simulink model "TosAeb"  (R2021a)
settings: Solver=FixedStepDiscrete  StartTime=0.0  StopTime=inf  FixedStep=0.01  RelTol=1e-3
undefined symbols (not defined in the file or in its folder): ACCModSts, AccSts1, AEB_ObjectMovingOrientation, AEB_ObjectMovingState, CN_BTCActive, CN_CurrentGearR, CN_CurrentGearR_DHT, CN_PTCActive, CN_VDCActive, CN_VSC_EspOff, CN_VSC_FCW_AEB_Error, CN_VSC_FCW_AEB_NoDispay, CN_VSC_FCW_AEB_PerfmDgdtn, CN_VSC_FCW_AEB_TempUnAvlbl, CN_VSC_VehStandstill, CNaccelAvoidMax, CNaccelAvoidMin, CNbrakePedalPress, CNcomfortStrLeftAccelMax, CNcomfortStrLeftAccelMin, CNcomfortStrRightAccelMax, CNcomfortStrRightAccelMin, CNDrvDoorOpen, CNDrvModeEco, CNDrvModeNorm, CNDrvModeOther, CNDrvModeSport, CNDrvSBRreminder, CNNull, CNobjInvalidID, CNPassDoorOpen, CNPassSBRreminder, CNPEBSAccelNegInf, CNPEBSAccelNull, CNPEBSTimeInf, CNPEBSTimeNull, CNSTOPMAX, CNSTOPNULL, CNsubjWidth, CNSwtOFF, CNSwtON, CNTTCMAX, CNTTCNULL, CNVehConfigAEBCycNone, CNVehConfigAEBNone, CNVehConfigAEBPedNone, CNVehConfigFCWNone, CNVehConfigJANone, CollisionSeverity, FALSE, FCWSnvty, JACycObjCalcInfoDefaultParams, JAPedObjCalcInfoDefaultParams, JAVehObjCalcInfoDefaultParams, latCycObjCalcInfoDefaultParams, latPedObjCalcInfoDefaultParams, longObjCalcInfoDefaultParams, ModSts, movingStatus, ObjectType ... (+1112)
libraries: simulink x3031, AEBSFunctionLib x50, Ta_Library x33, AEBSStateMachineLib x23, Mylibrary x12, simulink_extras x7, ObjSelect_lib x3, dspstat3 x2
(part 17 of 102)

== system TosAeb/FcwAebJa_Step_sys/ALG_AEBSystem/ALG_AEBController/AEB_StateMachineCalcApp/JaFctStsArb/BrkArb ==
  JA_vehAEBModSts : Inport
  JA_pedAEBModSts : Inport  Port=2
  JA_cycAEBModSts : Inport  Port=3
  JA_PTW_AEBModSts : Inport  Port=4
  BrkAborArb : Subsystem
  Bus Creator : BusCreator  Inputs=8
  Bus Creator1 : BusCreator  Inputs=4
  Bus Selector : BusSelector  OutputSignals=JA_vehAEBModSts,JA_pedAEBModSts,JA_cycAEBModSts,JA_PTW_AEBModSts
  Bus Selector1 : BusSelector  OutputSignals=JA_vehAEBModSts,JA_pedAEBModSts,JA_cycAEBModSts,JA_PTW_AEBModSts
  Bus Selector2 : BusSelector  OutputSignals=JA_vehAEBModSts,JA_PTW_AEBModSts,JA_cycAEBModSts,JA_pedAEBModSts
  JA_BrkActvArb : Subsystem
  JA_BrkModStsArbtrtn : Subsystem
  JA_BrkSuppArb : Subsystem
  JA_vehAEBModStsArb : Outport
  JA_PTW_AEBModStsArb : Outport  Port=2
  JA_cycAEBModStsArb : Outport  Port=3
  JA_pedAEBModStsArb : Outport  Port=4
  brkSuppAborCondArb : Outport  Port=5
  brkActvCond : Outport  Port=6
  wires:
  JA_BrkModStsArbtrtn/3(JA_cycAEBModStsArb) -> [JA_cycAEBModStsArb], JA_BrkActvArb/3(JA_cycAEBModStsArb)
  JA_BrkActvArb/1(JA_BrkActvCond) -> brkActvCond/1
  Bus Creator/1 -> brkSuppAborCondArb/1
  JA_BrkModStsArbtrtn/1(JA_vehAEBModStsArb) -> [JA_vehAEBModStsArb], JA_BrkActvArb/1(JA_vehAEBModStsArb)
  JA_BrkModStsArbtrtn/2(JA_PTW_AEBModStsArb) -> [JA_PTW_AEBModStsArb], JA_BrkActvArb/2(JA_PTW_AEBModStsArb)
  Bus Selector/1 -> JA_BrkSuppArb/1(JA_vehAEBModSts)  "<JA_vehAEBModSts>"
  Bus Selector/2 -> JA_BrkSuppArb/2(JA_pedAEBModSts)  "<JA_pedAEBModSts>"
  Bus Selector/3 -> JA_BrkSuppArb/3(JA_cycAEBModSts)  "<JA_cycAEBModSts>"
  Bus Selector/4 -> JA_BrkSuppArb/4(JA_PTW_AEBModSts)  "<JA_PTW_AEBModSts>"
  JA_PTW_AEBModSts/1 -> Bus Creator1/4  "JA_PTW_AEBModSts"
  JA_cycAEBModSts/1 -> Bus Creator1/3  "JA_cycAEBModSts"
  JA_pedAEBModSts/1 -> Bus Creator1/2  "JA_pedAEBModSts"
  JA_vehAEBModSts/1 -> Bus Creator1/1  "JA_vehAEBModSts"
  BrkAborArb/1(JA_vehAEBAborArb) -> Bus Creator/5, [JA_vehAEBAborArb]  "JA_vehAEBAborArb"
  BrkAborArb/2(JA_PTW_AEBAborArb) -> Bus Creator/6, [JA_PTW_AEBAborArb]  "JA_PTW_AEBAborArb"
  BrkAborArb/3(JA_cycAEBAborArb) -> Bus Creator/7, [JA_cycAEBAborArb]  "JA_cycAEBAborArb"
  BrkAborArb/4(JA_pedAEBAborArb) -> [JA_pedAEBAborArb], Bus Creator/8  "JA_pedAEBAborArb"
  Bus Selector1/1 -> BrkAborArb/1(JA_vehAEBModSts)  "<JA_vehAEBModSts>"
  Bus Selector1/2 -> BrkAborArb/2(JA_pedAEBModSts)  "<JA_pedAEBModSts>"
  Bus Selector1/3 -> BrkAborArb/3(JA_cycAEBModSts)  "<JA_cycAEBModSts>"
  Bus Selector1/4 -> BrkAborArb/4(JA_PTW_AEBModSts)  "<JA_PTW_AEBModSts>"
  JA_BrkSuppArb/1(JA_vehAEBSuppArb) -> Bus Creator/1  "JA_vehAEBSuppArb"
  JA_BrkSuppArb/2(JA_PTW_AEBSuppArb) -> Bus Creator/2  "JA_PTW_AEBSuppArb"
  JA_BrkSuppArb/3(JA_cycAEBSuppArb) -> Bus Creator/3  "JA_cycAEBSuppArb"
  JA_BrkSuppArb/4(JA_pedAEBSuppArb) -> Bus Creator/4  "JA_pedAEBSuppArb"
  [JA_vehAEBAborArb] -> JA_BrkModStsArbtrtn/5(JA_vehAEBAborArb)
  [JA_PTW_AEBAborArb] -> JA_BrkModStsArbtrtn/6(JA_PTW_AEBAborArb)
  [JA_cycAEBAborArb] -> JA_BrkModStsArbtrtn/7(JA_cycAEBAborArb)
  [JA_pedAEBAborArb] -> JA_BrkModStsArbtrtn/8(JA_pedAEBAborArb)
  Bus Creator1/1 -> Bus Selector2/1, Bus Selector1/1, Bus Selector/1
  JA_BrkModStsArbtrtn/4(JA_pedAEBModStsArb) -> JA_BrkActvArb/4(JA_pedAEBModStsArb), [JA_pedAEBModStsArb]
  [JA_vehAEBModStsArb] -> JA_vehAEBModStsArb/1
  [JA_PTW_AEBModStsArb] -> JA_PTW_AEBModStsArb/1
  [JA_pedAEBModStsArb] -> JA_pedAEBModStsArb/1
  [JA_cycAEBModStsArb] -> JA_cycAEBModStsArb/1
  Bus Selector2/1 -> JA_BrkModStsArbtrtn/1(JA_vehAEBModSts)  "<JA_vehAEBModSts>"
  Bus Selector2/2 -> JA_BrkModStsArbtrtn/2(JA_PTW_AEBModSts)  "<JA_PTW_AEBModSts>"
  Bus Selector2/3 -> JA_BrkModStsArbtrtn/3(JA_cycAEBModSts)  "<JA_cycAEBModSts>"
  Bus Selector2/4 -> JA_BrkModStsArbtrtn/4(JA_pedAEBModSts)  "<JA_pedAEBModSts>"

== system TosAeb/FcwAebJa_Step_sys/ALG_AEBSystem/ALG_AEBController/AEB_StateMachineCalcApp/JaFctStsArb/BrkReadyArb ==
  brkActvCond : Inport
  JA_vehPrefillModSts : Inport  Port=2
  JA_pedPrefillModSts : Inport  Port=3
  JA_cycPrefillModSts : Inport  Port=4
  JA_PTW_PrefillModSts : Inport  Port=5
  BrkAborArb : Subsystem
  BrkReadyActvArb : Subsystem
  BrkReadyModStsArbtrtn : Subsystem
  BrkReadySuppArb : Subsystem
  Bus Creator : BusCreator  Inputs=8
  Bus Creator1 : BusCreator  Inputs=5
  Bus Selector : BusSelector  OutputSignals=JA_vehPrefillModSts,JA_pedPrefillModSts,JA_cycPrefillModSts,JA_PTW_PrefillModSts,brkActvCond
  Bus Selector1 : BusSelector  OutputSignals=JA_vehPrefillModSts,JA_pedPrefillModSts,JA_cycPrefillModSts,JA_PTW_PrefillModSts,brkActvCond
  Bus Selector2 : BusSelector  OutputSignals=JA_vehPrefillModSts,JA_PTW_PrefillModSts,JA_cycPrefillModSts,JA_pedPrefillModSts
  Terminator : Terminator
  brkReadySuppAborCondArb : Outport
  JA_vehPrefillModStsArb : Outport  Port=2
  JA_PTW_PrefillModStsArb : Outport  Port=3
  JA_cycPrefillModStsArb : Outport  Port=4
  JA_pedPrefillModStsArb : Outport  Port=5
  wires:
  [JA_cycPrefillModStsArb] -> JA_cycPrefillModStsArb/1
  [JA_pedPrefillModStsArb] -> JA_pedPrefillModStsArb/1
  [JA_PTW_PrefillModStsArb] -> JA_PTW_PrefillModStsArb/1
  [JA_vehPrefillModStsArb] -> JA_vehPrefillModStsArb/1
  BrkReadyModStsArbtrtn/4(JA_pedPrefillModStsArb) -> BrkReadyActvArb/4(JA_pedPrefillModStsArb), [JA_pedPrefillModStsArb]
  Bus Creator1/1 -> Bus Selector/1, Bus Selector1/1, Bus Selector2/1
  Bus Selector2/1 -> BrkReadyModStsArbtrtn/1(JA_vehPrefillModSts)  "<JA_vehPrefillModSts>"
  Bus Selector2/3 -> BrkReadyModStsArbtrtn/3(JA_cycPrefillModSts)  "<JA_cycPrefillModSts>"
  [JA_pedPrefillAborArb] -> BrkReadyModStsArbtrtn/8(JA_pedPrefillAborArb)
  [JA_cycPrefillAborArb] -> BrkReadyModStsArbtrtn/7(JA_cycPrefillAborArb)
  [JA_PTW_PrefillAborArb] -> BrkReadyModStsArbtrtn/6(JA_PTW_PrefillAborArb)
  [JA_vehPrefillAborArb] -> BrkReadyModStsArbtrtn/5(JA_vehPrefillAborArb)
  BrkReadySuppArb/4(JA_pedPrefillSuppArb) -> Bus Creator/4  "JA_pedPrefillSuppArb"
  BrkReadySuppArb/3(JA_cycPrefillSuppArb) -> Bus Creator/3  "JA_cycPrefillSuppArb"
  BrkReadySuppArb/2(JA_PTW_PrefillSuppArb) -> Bus Creator/2  "JA_PTW_PrefillSuppArb"
  BrkReadySuppArb/1(JA_vehPrefillSuppArb) -> Bus Creator/1  "JA_vehPrefillSuppArb"
  Bus Selector1/4 -> BrkAborArb/4(JA_PTW_PrefillModSts)  "<JA_PTW_PrefillModSts>"
  Bus Selector1/3 -> BrkAborArb/3(JA_cycPrefillModSts)  "<JA_cycPrefillModSts>"
  Bus Selector1/2 -> BrkAborArb/2(JA_pedPrefillModSts)  "<JA_pedPrefillModSts>"
  Bus Selector1/1 -> BrkAborArb/1(JA_vehPrefillModSts)  "<JA_vehPrefillModSts>"
  BrkAborArb/4(JA_pedPrefillAborArb) -> Bus Creator/8, [JA_pedPrefillAborArb]  "JA_pedPrefillAborArb"
  BrkAborArb/3(JA_cycPrefillAborArb) -> [JA_cycPrefillAborArb], Bus Creator/7  "JA_cycPrefillAborArb"
  BrkAborArb/2(JA_PTW_PrefillAborArb) -> [JA_PTW_PrefillAborArb], Bus Creator/6  "JA_PTW_PrefillAborArb"
  BrkAborArb/1(JA_vehPrefillAborArb) -> [JA_vehPrefillAborArb], Bus Creator/5  "JA_vehPrefillAborArb"
  JA_vehPrefillModSts/1 -> Bus Creator1/1  "JA_vehPrefillModSts"
  JA_pedPrefillModSts/1 -> Bus Creator1/2  "JA_pedPrefillModSts"
  JA_cycPrefillModSts/1 -> Bus Creator1/3  "JA_cycPrefillModSts"
  JA_PTW_PrefillModSts/1 -> Bus Creator1/4  "JA_PTW_PrefillModSts"
  Bus Selector/5 -> BrkReadySuppArb/5(JA_BrkActvCond)  "<brkActvCond>"
  Bus Selector/4 -> BrkReadySuppArb/4(JA_PTW_PrefillModSts)  "<JA_PTW_PrefillModSts>"
  Bus Selector/3 -> BrkReadySuppArb/3(JA_cycPrefillModSts)  "<JA_cycPrefillModSts>"
  Bus Selector/2 -> BrkReadySuppArb/2(JA_pedPrefillModSts)  "<JA_pedPrefillModSts>"
  Bus Selector/1 -> BrkReadySuppArb/1(JA_vehPrefillModSts)  "<JA_vehPrefillModSts>"
  BrkReadyModStsArbtrtn/2(JA_PTW_PrefillModStsArb) -> BrkReadyActvArb/2(JA_PTW_PrefillModStsArb), [JA_PTW_PrefillModStsArb]
  BrkReadyModStsArbtrtn/1(JA_vehPrefillModStsArb) -> BrkReadyActvArb/1(JA_vehPrefillModStsArb), [JA_vehPrefillModStsArb]
  Bus Creator/1 -> brkReadySuppAborCondArb/1
  BrkReadyModStsArbtrtn/3(JA_cycPrefillModStsArb) -> BrkReadyActvArb/3(JA_cycPrefillModStsArb), [JA_cycPrefillModStsArb]
  brkActvCond/1 -> Bus Creator1/5  "brkActvCond"
  Bus Selector1/5 -> BrkAborArb/5(JA_BrkActvCond)  "<brkActvCond>"
  BrkReadyActvArb/1(JA_BrkActvCond) -> Terminator/1
  Bus Selector2/4 -> BrkReadyModStsArbtrtn/4(JA_pedPrefillModSts)  "<JA_pedPrefillModSts>"
  Bus Selector2/2 -> BrkReadyModStsArbtrtn/2(JA_PTW_PrefillModSts)  "<JA_PTW_PrefillModSts>"

== system TosAeb/FcwAebJa_Step_sys/ALG_AEBSystem/ALG_AEBController/AEB_StateMachineCalcApp/LatAEBStateMachine/cycAEBfullStateMachine ==
  inputSignal : Inport
  AEBFuncFaultMap : Subsystem
  AEBStateMachine : AEBSStateMachineLib/AEBStateMachine  SystemSampleTime=-1  VariantActivationTime=update diagram  Latency=0  AutoFrameSizeCalculation=off
  ActivationCondCalc : Subsystem
  ActiveStateAborCondCalc : Subsystem
  Bus Selector : BusSelector  OutputSignals=AVHStsActv,vehStateCalcInfo.VSC_FCW_AEB_FctSts.FCW_AEB_PedFctSts,vehStateCalcInfo.VSC_AEBFuncSwtSts.AEB_PedResp,vehStateCalcInfo.VSCVehStandStill
  Bus Selector1 : BusSelector  OutputSignals=CycFuncSuppAborCond.cycAEBfullSuppCond,latFctStsArbSuppAborCond.brkSuppAborCondArb.cycAEBfullSuppArb,FuncImpSuppAborCond.brkFuncImpSuppAborCond.brkFuncImpSuppCond.cycAEBfullFuncImpSuppCond
  Bus Selector2 : BusSelector  OutputSignals=CycFuncSuppAborCond.cycAEBfullAborCondNoStandstill,latFctStsArbSuppAborCond.brkSuppAborCondArb.cycAEBfullAborArb,FuncImpSuppAborCond.brkFuncImpSuppAborCond.brkFuncImpAborCond.cycAEBfullFuncImpAborCond,FuncManeuAborCond.latBrkManeuAborCond
  Bus Selector3 : BusSelector  OutputSignals=CycFuncSuppAborCond.cycAEBfullAborCondStandstill,latFctStsArbSuppAborCond.brkSuppAborCondArb.cycAEBfullAborArb,FuncImpSuppAborCond.brkFuncImpSuppAborCond.brkFuncImpAborCond.cycAEBfullFuncImpAborCond,FuncManeuAborCond.latBrkManeuAborCond
  Constant : Constant  Value=5
  Constant1 : Constant  Value=P_AEB_FuncStatus_CycAEBfullStandstillWorkTime
  Constant2 : Constant  Value=Ts
  StandstillAborCondCalc : Subsystem
  cycAEBfullRawModSts : Outport
  cycAEBfullModSts : Outport  Port=2
  cycAEBfullFuncActiveTime : Outport  Port=3
  wires:
  inputSignal/1 -> Bus Selector3/1, Bus Selector2/1, Bus Selector1/1, Bus Selector/1
  Bus Selector2/2 -> ActiveStateAborCondCalc/2(cycAEBfullAborArb)  "<cycAEBfullAborArb>"
  Bus Selector2/3 -> ActiveStateAborCondCalc/3(cycAEBfullFuncImpAborCond)  "<cycAEBfullFuncImpAborCond>"
  Bus Selector1/2 -> ActivationCondCalc/2(cycAEBfullSuppArb)  "<cycAEBfullSuppArb>"
  Bus Selector1/3 -> ActivationCondCalc/3(cycAEBfullFuncImpSuppCond)  "<cycAEBfullFuncImpSuppCond>"
  Bus Selector2/1 -> ActiveStateAborCondCalc/1(cycAEBfullAborCondNoStandstill)  "<cycAEBfullAborCondNoStandstill>"
  Bus Selector1/1 -> ActivationCondCalc/1(cycAEBfullSuppCond)  "<cycAEBfullSuppCond>"
  Bus Selector2/4 -> ActiveStateAborCondCalc/4(latBrkManeuAborCond)  "<latBrkManeuAborCond>"
  Bus Selector3/1 -> StandstillAborCondCalc/1(cycAEBfullAborCondStandstill)  "<cycAEBfullAborCondStandstill>"
  Bus Selector3/2 -> StandstillAborCondCalc/2(cycAEBfullAborArb)  "<cycAEBfullAborArb>"
  Bus Selector3/3 -> StandstillAborCondCalc/3(cycAEBfullFuncImpAborCond)  "<cycAEBfullFuncImpAborCond>"
  Bus Selector3/4 -> StandstillAborCondCalc/4(latBrkManeuAborCond)  "<latBrkManeuAborCond>"
  AEBStateMachine/3 -> cycAEBfullFuncActiveTime/1  "cycAEBfullFuncActiveTime"
  AEBStateMachine/2 -> cycAEBfullModSts/1  "cycAEBfullModSts"
  AEBStateMachine/1 -> cycAEBfullRawModSts/1
  Constant2/1 -> AEBStateMachine/8
  Constant/1 -> AEBStateMachine/9
  Constant1/1 -> AEBStateMachine/10
  Bus Selector/2 -> AEBFuncFaultMap/1(FCW_AEB_VehFctSts)  "<FCW_AEB_PedFctSts>"
  Bus Selector/3 -> AEBStateMachine/3  "<AEB_PedResp>"
  Bus Selector/1 -> AEBStateMachine/1  "<AVHStsActv>"
  Bus Selector/4 -> AEBStateMachine/4  "<VSCVehStandStill>"
  ActivationCondCalc/1(ActivationCondFulfilled) -> AEBStateMachine/5
  ActiveStateAborCondCalc/1(ActiveStateAborCondFulfilled) -> AEBStateMachine/6
  StandstillAborCondCalc/1(ActiveStateAborCondFulfilled) -> AEBStateMachine/7
  AEBFuncFaultMap/1(Fault) -> AEBStateMachine/2

== system TosAeb/FcwAebJa_Step_sys/ALG_AEBSystem/ALG_AEBController/AEB_StateMachineCalcApp/LatAEBStateMachine/cycAEBpartStateMachine ==
  inputSignal : Inport
  AEBFuncFaultMap : Subsystem
  AEBStateMachine : AEBSStateMachineLib/AEBStateMachine  SystemSampleTime=-1  VariantActivationTime=update diagram  Latency=0  AutoFrameSizeCalculation=off
  ActivationCondCalc : Subsystem
  ActiveStateAborCondCalc : Subsystem
  Bus Selector : BusSelector  OutputSignals=AVHStsActv,vehStateCalcInfo.VSC_FCW_AEB_FctSts.FCW_AEB_PedFctSts,vehStateCalcInfo.VSC_AEBFuncSwtSts.AEB_PedResp,vehStateCalcInfo.VSCVehStandStill
  Bus Selector1 : BusSelector  OutputSignals=CycFuncSuppAborCond.cycAEBpartSuppCond,latFctStsArbSuppAborCond.brkSuppAborCondArb.cycAEBpartSuppArb,FuncImpSuppAborCond.brkFuncImpSuppAborCond.brkFuncImpSuppCond.cycAEBpartFuncImpSuppCond
  Bus Selector2 : BusSelector  OutputSignals=CycFuncSuppAborCond.cycAEBpartAborCondNoStandstill,latFctStsArbSuppAborCond.brkSuppAborCondArb.cycAEBpartAborArb,FuncImpSuppAborCond.brkFuncImpSuppAborCond.brkFuncImpAborCond.cycAEBpartFuncImpAborCond,FuncManeuAborCond.latBrkManeuAborCond
  Bus Selector3 : BusSelector  OutputSignals=CycFuncSuppAborCond.cycAEBpartAborCondStandstill,latFctStsArbSuppAborCond.brkSuppAborCondArb.cycAEBpartAborArb,FuncImpSuppAborCond.brkFuncImpSuppAborCond.brkFuncImpAborCond.cycAEBpartFuncImpAborCond,FuncManeuAborCond.latBrkManeuAborCond
  Constant : Constant  Value=5
  Constant1 : Constant  Value=P_AEB_FuncStatus_CycAEBpartStandstillWorkTime
  Constant2 : Constant  Value=Ts
  StandstillAborCondCalc : Subsystem
  cycAEBpartRawModSts : Outport
  cycAEBpartModSts : Outport  Port=2
  cycAEBpartFuncActiveTime : Outport  Port=3
  wires:
  inputSignal/1 -> Bus Selector3/1, Bus Selector2/1, Bus Selector1/1, Bus Selector/1
  Bus Selector2/2 -> ActiveStateAborCondCalc/2(cycAEBpartAborArb)  "<cycAEBpartAborArb>"
  Bus Selector2/3 -> ActiveStateAborCondCalc/3(cycAEBpartFuncImpAborCond)  "<cycAEBpartFuncImpAborCond>"
  Bus Selector1/2 -> ActivationCondCalc/2(cycAEBpartSuppArb)  "<cycAEBpartSuppArb>"
  Bus Selector1/3 -> ActivationCondCalc/3(cycAEBpartFuncImpSuppCond)  "<cycAEBpartFuncImpSuppCond>"
  Bus Selector2/1 -> ActiveStateAborCondCalc/1(cycAEBpartAborCondNoStandstill)  "<cycAEBpartAborCondNoStandstill>"
  Bus Selector1/1 -> ActivationCondCalc/1(cycAEBpartSuppCond)  "<cycAEBpartSuppCond>"
  Bus Selector2/4 -> ActiveStateAborCondCalc/4(latBrkManeuAborCond)  "<latBrkManeuAborCond>"
  Bus Selector3/1 -> StandstillAborCondCalc/1(cycAEBpartAborCondStandstill)  "<cycAEBpartAborCondStandstill>"
  Bus Selector3/2 -> StandstillAborCondCalc/2(cycAEBpartAborArb)  "<cycAEBpartAborArb>"
  Bus Selector3/3 -> StandstillAborCondCalc/3(cycAEBpartFuncImpAborCond)  "<cycAEBpartFuncImpAborCond>"
  Bus Selector3/4 -> StandstillAborCondCalc/4(latBrkManeuAborCond)  "<latBrkManeuAborCond>"
  AEBStateMachine/3 -> cycAEBpartFuncActiveTime/1  "cycAEBpartFuncActiveTime"
  AEBStateMachine/2 -> cycAEBpartModSts/1  "cycAEBpartModSts"
  AEBStateMachine/1 -> cycAEBpartRawModSts/1
  Bus Selector/2 -> AEBFuncFaultMap/1(FCW_AEB_VehFctSts)  "<FCW_AEB_PedFctSts>"
  Bus Selector/3 -> AEBStateMachine/3  "<AEB_PedResp>"
  Bus Selector/1 -> AEBStateMachine/1  "<AVHStsActv>"
  Bus Selector/4 -> AEBStateMachine/4  "<VSCVehStandStill>"
  Constant2/1 -> AEBStateMachine/8
  Constant/1 -> AEBStateMachine/9
  Constant1/1 -> AEBStateMachine/10
  ActivationCondCalc/1(ActivationCondFulfilled) -> AEBStateMachine/5
  ActiveStateAborCondCalc/1(ActiveStateAborCondFulfilled) -> AEBStateMachine/6
  StandstillAborCondCalc/1(ActiveStateAborCondFulfilled) -> AEBStateMachine/7
  AEBFuncFaultMap/1(Fault) -> AEBStateMachine/2

== system TosAeb/FcwAebJa_Step_sys/ALG_AEBSystem/ALG_AEBController/AEB_StateMachineCalcApp/LatAEBStateMachine/cycPrefillStateMachine ==
  inputSignal : Inport
  AEBFuncFaultMap : Subsystem
  AEBStateMachine : AEBSStateMachineLib/AEBStateMachine  SystemSampleTime=-1  VariantActivationTime=update diagram  Latency=0  AutoFrameSizeCalculation=off
  ActivationCondCalc : Subsystem
  ActiveStateAborCondCalc : Subsystem
  Bus Selector : BusSelector  OutputSignals=vehStateCalcInfo.VSC_FCW_AEB_FctSts.FCW_AEB_PedFctSts,vehStateCalcInfo.VSC_AEBFuncSwtSts.AEB_PedResp
  Bus Selector1 : BusSelector  OutputSignals=CycFuncSuppAborCond.cycPrefillSuppCond,latFctStsArbSuppAborCond.brkReadySuppAborCond.cycPrefillSuppArb,FuncImpSuppAborCond.brkReadyFuncImpSuppAborCond.brkReadyFuncImpSuppCond.cycPrefillFuncImpSuppCond
  Bus Selector2 : BusSelector  OutputSignals=CycFuncSuppAborCond.cycPrefillAborCond,latFctStsArbSuppAborCond.brkReadySuppAborCond.cycPrefillAborArb,FuncImpSuppAborCond.brkReadyFuncImpSuppAborCond.brkReadyFuncImpAborCond.cycPrefillFuncImpAborCond,FuncManeuAborCond.ABPFuncManeuAborCond
  Constant : Constant  Value=5
  Constant1 : Constant  Value=P_AEB_FuncStatus_CycPrefillStandstillWorkTime
  Constant2 : Constant  Value=Ts
  Constant3 : Constant  Value=FALSE
  Constant4 : Constant  Value=FALSE
  Constant5 : Constant  Value=FALSE
  cycPrefillRawModSts : Outport
  cycPrefillModSts : Outport  Port=2
  cycPrefillFuncActiveTime : Outport  Port=3
  wires:
  Bus Selector1/2 -> ActivationCondCalc/2(cycPrefillSuppArb)  "<cycPrefillSuppArb>"
  Bus Selector1/1 -> ActivationCondCalc/1(cycPrefillSuppCond)  "<cycPrefillSuppCond>"
  Bus Selector2/1 -> ActiveStateAborCondCalc/1(cycPrefillAborCond)  "<cycPrefillAborCond>"
  Bus Selector1/3 -> ActivationCondCalc/3(cycPrefillFuncImpSuppCond)  "<cycPrefillFuncImpSuppCond>"
  Bus Selector2/3 -> ActiveStateAborCondCalc/3(cycPrefillFuncImpAborCond)  "<cycPrefillFuncImpAborCond>"
  Bus Selector2/4 -> ActiveStateAborCondCalc/4(ABPFuncManeuAborCond)  "<ABPFuncManeuAborCond>"
  Bus Selector2/2 -> ActiveStateAborCondCalc/2(cycPrefillAborArb)  "<cycPrefillAborArb>"
  inputSignal/1 -> Bus Selector2/1, Bus Selector1/1, Bus Selector/1
  AEBStateMachine/3 -> cycPrefillFuncActiveTime/1  "cycPrefillFuncActiveTime"
  AEBStateMachine/2 -> cycPrefillModSts/1  "cycPrefillModSts"
  AEBStateMachine/1 -> cycPrefillRawModSts/1
  Constant5/1 -> AEBStateMachine/1
  Constant4/1 -> AEBStateMachine/4
  Bus Selector/1 -> AEBFuncFaultMap/1(FCW_AEB_VehFctSts)  "<FCW_AEB_PedFctSts>"
  Bus Selector/2 -> AEBStateMachine/3  "<AEB_PedResp>"
  Constant3/1 -> AEBStateMachine/7
  Constant2/1 -> AEBStateMachine/8
  Constant/1 -> AEBStateMachine/9
  Constant1/1 -> AEBStateMachine/10
  ActivationCondCalc/1(ActivationCondFulfilled) -> AEBStateMachine/5
  ActiveStateAborCondCalc/1(ActiveStateAborCondFulfilled) -> AEBStateMachine/6
  AEBFuncFaultMap/1(Fault) -> AEBStateMachine/2

== system TosAeb/FcwAebJa_Step_sys/ALG_AEBSystem/ALG_AEBController/AEB_StateMachineCalcApp/LatAEBStateMachine/pedABAStateMachine ==
  inputSignal : Inport
  AEBFuncFaultMap : Subsystem
  AEBStateMachine : AEBSStateMachineLib/AEBStateMachine  SystemSampleTime=-1  VariantActivationTime=update diagram  Latency=0  AutoFrameSizeCalculation=off
  ActivationCondCalc : Subsystem
  ActiveStateAborCondCalc : Subsystem
  Bus Selector : BusSelector  OutputSignals=vehStateCalcInfo.VSC_FCW_AEB_FctSts.FCW_AEB_PedFctSts,vehStateCalcInfo.VSC_AEBFuncSwtSts.AEB_PedResp
  Bus Selector1 : BusSelector  OutputSignals=PedFuncSuppAborCond.pedABASuppCond,latFctStsArbSuppAborCond.brkReadySuppAborCond.pedABASuppArb,FuncImpSuppAborCond.brkReadyFuncImpSuppAborCond.brkReadyFuncImpSuppCond.pedABAFuncImpSuppCond
  Bus Selector2 : BusSelector  OutputSignals=PedFuncSuppAborCond.pedABAAborCond,latFctStsArbSuppAborCond.brkReadySuppAborCond.pedABAAborArb,FuncImpSuppAborCond.brkReadyFuncImpSuppAborCond.brkReadyFuncImpAborCond.pedABAFuncImpAborCond,FuncManeuAborCond.ABAFuncManeuAborCond
  Constant : Constant  Value=256
  Constant1 : Constant  Value=P_AEB_FuncStatus_PedABAStandstillWorkTime
  Constant2 : Constant  Value=Ts
  Constant3 : Constant  Value=FALSE
  Constant4 : Constant  Value=FALSE
  Constant5 : Constant  Value=FALSE
  pedABARawModSts : Outport
  pedABAModSts : Outport  Port=2
  pedABAFuncActiveTime : Outport  Port=3
  wires:
  Bus Selector1/2 -> ActivationCondCalc/2(pedABASuppArb)  "<pedABASuppArb>"
  Bus Selector1/1 -> ActivationCondCalc/1(pedABASuppCond)  "<pedABASuppCond>"
  Bus Selector2/1 -> ActiveStateAborCondCalc/1(pedABAAborCond)  "<pedABAAborCond>"
  Bus Selector1/3 -> ActivationCondCalc/3(pedABAFuncImpSuppCond)  "<pedABAFuncImpSuppCond>"
  Bus Selector2/3 -> ActiveStateAborCondCalc/3(pedABAFuncImpAborCond)  "<pedABAFuncImpAborCond>"
  ActivationCondCalc/1(ActivationCondFulfilled) -> AEBStateMachine/5
  ActiveStateAborCondCalc/1(ActiveStateAborCondFulfilled) -> AEBStateMachine/6
  Bus Selector2/4 -> ActiveStateAborCondCalc/4(ABAFuncManeuAborCond)  "<ABAFuncManeuAborCond>"
  Bus Selector2/2 -> ActiveStateAborCondCalc/2(pedABAAborArb)  "<pedABAAborArb>"
  inputSignal/1 -> Bus Selector2/1, Bus Selector1/1, Bus Selector/1
  AEBStateMachine/1 -> pedABARawModSts/1
  AEBStateMachine/2 -> pedABAModSts/1  "pedABAModSts"
  AEBStateMachine/3 -> pedABAFuncActiveTime/1  "pedABAFuncActiveTime"
  Constant3/1 -> AEBStateMachine/7
  Constant2/1 -> AEBStateMachine/8
  Constant/1 -> AEBStateMachine/9
  Constant1/1 -> AEBStateMachine/10
  Constant5/1 -> AEBStateMachine/1
  Constant4/1 -> AEBStateMachine/4
  Bus Selector/1 -> AEBFuncFaultMap/1(FCW_AEB_VehFctSts)  "<FCW_AEB_PedFctSts>"
  Bus Selector/2 -> AEBStateMachine/3  "<AEB_PedResp>"
  AEBFuncFaultMap/1(Fault) -> AEBStateMachine/2

== system TosAeb/FcwAebJa_Step_sys/ALG_AEBSystem/ALG_AEBController/AEB_StateMachineCalcApp/LatAEBStateMachine/pedAEBfullStateMachine ==
  inputSignal : Inport
  AEBFuncFaultMap : Subsystem
  AEBStateMachine : AEBSStateMachineLib/AEBStateMachine  SystemSampleTime=-1  VariantActivationTime=update diagram  Latency=0  AutoFrameSizeCalculation=off
  ActivationCondCalc : Subsystem
  ActiveStateAborCondCalc : Subsystem
  Bus Selector : BusSelector  OutputSignals=AVHStsActv,vehStateCalcInfo.VSC_FCW_AEB_FctSts.FCW_AEB_PedFctSts,vehStateCalcInfo.VSC_AEBFuncSwtSts.AEB_PedResp,vehStateCalcInfo.VSCVehStandStill
  Bus Selector1 : BusSelector  OutputSignals=PedFuncSuppAborCond.pedAEBfullSuppCond,latFctStsArbSuppAborCond.brkSuppAborCondArb.pedAEBfullSuppArb,FuncImpSuppAborCond.brkFuncImpSuppAborCond.brkFuncImpSuppCond.pedAEBfullFuncImpSuppCond
  Bus Selector2 : BusSelector  OutputSignals=PedFuncSuppAborCond.pedAEBfullAborCondNoStandstill,latFctStsArbSuppAborCond.brkSuppAborCondArb.pedAEBfullAborArb,FuncImpSuppAborCond.brkFuncImpSuppAborCond.brkFuncImpAborCond.pedAEBfullFuncImpAborCond,FuncManeuAborCond.latBrkManeuAborCond
  Bus Selector3 : BusSelector  OutputSignals=PedFuncSuppAborCond.pedAEBfullAborCondStandstill,latFctStsArbSuppAborCond.brkSuppAborCondArb.pedAEBfullAborArb,FuncImpSuppAborCond.brkFuncImpSuppAborCond.brkFuncImpAborCond.pedAEBfullFuncImpAborCond,FuncManeuAborCond.latBrkManeuAborCond
  Constant : Constant  Value=5
  Constant1 : Constant  Value=P_AEB_FuncStatus_PedAEBfullStandstillWorkTime
  Constant2 : Constant  Value=Ts
  StandstillAborCondCalc : Subsystem
  pedAEBfullRawModSts : Outport
  pedAEBfullModSts : Outport  Port=2
  pedAEBfullFuncActiveTime : Outport  Port=3
  wires:
  inputSignal/1 -> Bus Selector3/1, Bus Selector2/1, Bus Selector1/1, Bus Selector/1
  Bus Selector1/2 -> ActivationCondCalc/2(pedAEBfullSuppArb)  "<pedAEBfullSuppArb>"
  Bus Selector1/1 -> ActivationCondCalc/1(pedAEBfullSuppCond)  "<pedAEBfullSuppCond>"
  Bus Selector2/1 -> ActiveStateAborCondCalc/1(pedAEBfullAborCondNoStandstill)  "<pedAEBfullAborCondNoStandstill>"
  Bus Selector1/3 -> ActivationCondCalc/3(pedAEBfullFuncImpSuppCond)  "<pedAEBfullFuncImpSuppCond>"
  Bus Selector2/2 -> ActiveStateAborCondCalc/2(pedAEBfullAborArb)  "<pedAEBfullAborArb>"
  Bus Selector2/3 -> ActiveStateAborCondCalc/3(pedAEBfullFuncImpAborCond)  "<pedAEBfullFuncImpAborCond>"
  Bus Selector2/4 -> ActiveStateAborCondCalc/4(latBrkManeuAborCond)  "<latBrkManeuAborCond>"
  Bus Selector3/4 -> StandstillAborCondCalc/4(latBrkManeuAborCond)  "<latBrkManeuAborCond>"
  Bus Selector3/3 -> StandstillAborCondCalc/3(pedAEBfullFuncImpAborCond)  "<pedAEBfullFuncImpAborCond>"
  Bus Selector3/2 -> StandstillAborCondCalc/2(pedAEBfullAborArb)  "<pedAEBfullAborArb>"
  Bus Selector3/1 -> StandstillAborCondCalc/1(pedAEBfullAborCondStandstill)  "<pedAEBfullAborCondStandstill>"
  AEBStateMachine/2 -> pedAEBfullModSts/1  "pedAEBfullModSts"
  AEBStateMachine/3 -> pedAEBfullFuncActiveTime/1  "pedAEBfullFuncActiveTime"
  AEBStateMachine/1 -> pedAEBfullRawModSts/1
  Bus Selector/2 -> AEBFuncFaultMap/1(FCW_AEB_VehFctSts)  "<FCW_AEB_PedFctSts>"
  Bus Selector/3 -> AEBStateMachine/3  "<AEB_PedResp>"
  Bus Selector/1 -> AEBStateMachine/1  "<AVHStsActv>"
  Bus Selector/4 -> AEBStateMachine/4  "<VSCVehStandStill>"
  Constant2/1 -> AEBStateMachine/8
  Constant/1 -> AEBStateMachine/9
  Constant1/1 -> AEBStateMachine/10
  ActivationCondCalc/1(ActivationCondFulfilled) -> AEBStateMachine/5
  ActiveStateAborCondCalc/1(ActiveStateAborCondFulfilled) -> AEBStateMachine/6
  StandstillAborCondCalc/1(ActiveStateAborCondFulfilled) -> AEBStateMachine/7
  AEBFuncFaultMap/1(Fault) -> AEBStateMachine/2

== system TosAeb/FcwAebJa_Step_sys/ALG_AEBSystem/ALG_AEBController/AEB_StateMachineCalcApp/LatAEBStateMachine/pedPreWarningStateMachine ==
  inputSignal : Inport
  AEBFuncFaultMap : Subsystem
  AEBStateMachine : AEBSStateMachineLib/AEBStateMachine  SystemSampleTime=-1  VariantActivationTime=update diagram  Latency=0  AutoFrameSizeCalculation=off
  ActivationCondCalc : Subsystem
  ActiveStateAborCondCalc : Subsystem
  Bus Selector : BusSelector  OutputSignals=vehStateCalcInfo.VSC_FCW_AEB_FctSts.FCW_AEB_PedFctSts,vehStateCalcInfo.VSC_AEBFuncSwtSts.AEB_PedResp
  Bus Selector1 : BusSelector  OutputSignals=PedFuncSuppAborCond.pedPreWarningSuppCond,latFctStsArbSuppAborCond.warningSuppAborCond.pedPrewarningSuppArb,FuncImpSuppAborCond.warningFuncImpSuppAborCond.warningFuncImpSuppCond.pedPreWarningFuncImpSuppCond
  Bus Selector2 : BusSelector  OutputSignals=PedFuncSuppAborCond.pedPreWarningAborCond,latFctStsArbSuppAborCond.warningSuppAborCond.pedPreWarningAborArb,FuncImpSuppAborCond.warningFuncImpSuppAborCond.warningFuncImpAborCond.pedPreWarningFuncImpAborCond
  Constant : Constant  Value=256
  Constant1 : Constant  Value=P_AEB_FuncStatus_PedPreWarningStandstillWorkTime
  Constant2 : Constant  Value=Ts
  Constant3 : Constant  Value=FALSE
  Constant4 : Constant  Value=FALSE
  Constant5 : Constant  Value=FALSE
  pedPreWarningRawModSts : Outport
  pedPreWarningModSts : Outport  Port=2
  pedPreWarningFuncActiveTime : Outport  Port=3
  wires:
  AEBStateMachine/2 -> pedPreWarningModSts/1  "pedPreWarningModSts"
  Bus Selector1/3 -> ActivationCondCalc/3(pedPreWarningFuncImpSuppCond)  "<pedPreWarningFuncImpSuppCond>"
  Bus Selector2/1 -> ActiveStateAborCondCalc/1(pedPreWarningAborCond)  "<pedPreWarningAborCond>"
  Bus Selector1/1 -> ActivationCondCalc/1(pedPreWarningSuppCond)  "<pedPreWarningSuppCond>"
  Bus Selector1/2 -> ActivationCondCalc/2(pedPreWarningSuppArb)  "<pedPrewarningSuppArb>"
  inputSignal/1 -> Bus Selector2/1, Bus Selector1/1, Bus Selector/1
  Bus Selector2/2 -> ActiveStateAborCondCalc/2(pedPreWarningAborArb)  "<pedPreWarningAborArb>"
  Bus Selector2/3 -> ActiveStateAborCondCalc/3(pedPreWarningFuncImpAborCond)  "<pedPreWarningFuncImpAborCond>"
  AEBStateMachine/3 -> pedPreWarningFuncActiveTime/1  "pedPreWarningFuncActiveTime"
  AEBStateMachine/1 -> pedPreWarningRawModSts/1
  Constant3/1 -> AEBStateMachine/7
  Constant2/1 -> AEBStateMachine/8
  Constant/1 -> AEBStateMachine/9
  Constant1/1 -> AEBStateMachine/10
  Constant4/1 -> AEBStateMachine/4
  Bus Selector/1 -> AEBFuncFaultMap/1(FCW_AEB_VehFctSts)  "<FCW_AEB_PedFctSts>"
  Bus Selector/2 -> AEBStateMachine/3  "<AEB_PedResp>"
  Constant5/1 -> AEBStateMachine/1
  ActivationCondCalc/1(ActivationCondFulfilled) -> AEBStateMachine/5
  ActiveStateAborCondCalc/1(ActiveStateAborCondFulfilled) -> AEBStateMachine/6
  AEBFuncFaultMap/1(Fault) -> AEBStateMachine/2

== system TosAeb/FcwAebJa_Step_sys/ALG_AEBSystem/ALG_AEBController/AEB_StateMachineCalcApp/LatAEBStateMachine/pedPrefillStateMachine ==
  inputSignal : Inport
  AEBFuncFaultMap : Subsystem
  AEBStateMachine : AEBSStateMachineLib/AEBStateMachine  SystemSampleTime=-1  VariantActivationTime=update diagram  Latency=0  AutoFrameSizeCalculation=off
  ActivationCondCalc : Subsystem
  ActiveStateAborCondCalc : Subsystem
  Bus Selector : BusSelector  OutputSignals=vehStateCalcInfo.VSC_FCW_AEB_FctSts.FCW_AEB_PedFctSts,vehStateCalcInfo.VSC_AEBFuncSwtSts.AEB_PedResp
  Bus Selector1 : BusSelector  OutputSignals=PedFuncSuppAborCond.pedPrefillSuppCond,latFctStsArbSuppAborCond.brkReadySuppAborCond.pedPrefillSuppArb,FuncImpSuppAborCond.brkReadyFuncImpSuppAborCond.brkReadyFuncImpSuppCond.pedPrefillFuncImpSuppCond
  Bus Selector2 : BusSelector  OutputSignals=PedFuncSuppAborCond.pedPrefillAborCond,latFctStsArbSuppAborCond.brkReadySuppAborCond.pedPrefillAborArb,FuncImpSuppAborCond.brkReadyFuncImpSuppAborCond.brkReadyFuncImpAborCond.pedPrefillFuncImpAborCond,FuncManeuAborCond.ABPFuncManeuAborCond
  Constant : Constant  Value=256
  Constant1 : Constant  Value=P_AEB_FuncStatus_PedPrefillStandstillWorkTime
  Constant2 : Constant  Value=Ts
  Constant3 : Constant  Value=FALSE
  Constant4 : Constant  Value=FALSE
  Constant5 : Constant  Value=FALSE
  pedPrefillRawModSts : Outport
  pedPrefillModSts : Outport  Port=2
  pedPrefillFuncActiveTime : Outport  Port=3
  wires:
  AEBStateMachine/2 -> pedPrefillModSts/1  "pedPrefillModSts"
  inputSignal/1 -> Bus Selector/1, Bus Selector2/1, Bus Selector1/1
  Bus Selector1/2 -> ActivationCondCalc/2(pedPrefillSuppArb)  "<pedPrefillSuppArb>"
  Bus Selector1/1 -> ActivationCondCalc/1(pedPrefillSuppCond)  "<pedPrefillSuppCond>"
  Bus Selector1/3 -> ActivationCondCalc/3(pedPrefillFuncImpSuppCond)  "<pedPrefillFuncImpSuppCond>"
  Bus Selector2/1 -> ActiveStateAborCondCalc/1(pedPrefillAborCond)  "<pedPrefillAborCond>"
  Bus Selector2/2 -> ActiveStateAborCondCalc/2(pedPrefillAborArb)  "<pedPrefillAborArb>"
  Bus Selector2/3 -> ActiveStateAborCondCalc/3(pedPrefillFuncImpAborCond)  "<pedPrefillFuncImpAborCond>"
  Bus Selector2/4 -> ActiveStateAborCondCalc/4(vehBrkManeuAborCond)  "<ABPFuncManeuAborCond>"
  AEBStateMachine/3 -> pedPrefillFuncActiveTime/1  "pedPrefillFuncActiveTime"
  AEBStateMachine/1 -> pedPrefillRawModSts/1
  Constant3/1 -> AEBStateMachine/7
  Constant2/1 -> AEBStateMachine/8
  Constant/1 -> AEBStateMachine/9
  Constant1/1 -> AEBStateMachine/10
  Constant5/1 -> AEBStateMachine/1
  Constant4/1 -> AEBStateMachine/4
  Bus Selector/1 -> AEBFuncFaultMap/1(FCW_AEB_VehFctSts)  "<FCW_AEB_PedFctSts>"
  Bus Selector/2 -> AEBStateMachine/3  "<AEB_PedResp>"
  ActivationCondCalc/1(ActivationCondFulfilled) -> AEBStateMachine/5
  ActiveStateAborCondCalc/1(ActiveStateAborCondFulfilled) -> AEBStateMachine/6
  AEBFuncFaultMap/1(Fault) -> AEBStateMachine/2

== system TosAeb/FcwAebJa_Step_sys/ALG_AEBSystem/ALG_AEBController/AEB_StateMachineCalcApp/LatFctStsArb/BrkArb ==
  vehAEBModSts : Inport
  BrkAborArb : Subsystem
  BrkActvArb : Subsystem
  BrkModStsArb : Subsystem
  BrkSuppArb : Subsystem
  Bus Creator : BusCreator  Inputs=6
  brkActvCond : Outport
  cycAEBpartModStsArb : Outport  Port=2
  cycAEBfullModStsArb : Outport  Port=3
  pedAEBfullModStsArb : Outport  Port=4
  brkSuppAborCondArb : Outport  Port=5
  wires:
  BrkModStsArb/2(cycAEBfullModStsArb) -> [cycAEBfullModStsArb], BrkActvArb/2(cycAEBfullModStsArb)
  BrkModStsArb/1(cycAEBpartModStsArb) -> BrkActvArb/1(cycAEBpartModStsArb), [cycAEBpartModStsArb]
  BrkSuppArb/1(cycAEBpartSuppArb) -> Bus Creator/1  "cycAEBpartSuppArb"
  [pedAEBfullAborArb] -> BrkModStsArb/4(pedAEBfullAborArb)
  [cycAEBpartAborArb] -> BrkModStsArb/2(cycAEBpartAborArb)
  BrkAborArb/1(cycAEBpartAborArb) -> [cycAEBpartAborArb], Bus Creator/4  "cycAEBpartAborArb"
  Bus Creator/1 -> brkSuppAborCondArb/1
  BrkSuppArb/3(pedAEBfullSuppArb) -> Bus Creator/3  "pedAEBfullSuppArb"
  vehAEBModSts/1 -> BrkModStsArb/1(vehAEBModSts), BrkAborArb/1(vehAEBModSts), BrkSuppArb/1(vehAEBModSts)
  BrkAborArb/2(cycAEBfullAborArb) -> [cycAEBfullAborArb], Bus Creator/5  "cycAEBfullAborArb"
  BrkActvArb/1(brkActvCond) -> brkActvCond/1
  BrkSuppArb/2(cycAEBfullSuppArb) -> Bus Creator/2  "cycAEBfullSuppArb"
  [cycAEBfullAborArb] -> BrkModStsArb/3(cycAEBfullAborArb)
  BrkAborArb/3(pedAEBfullAborArb) -> Bus Creator/6, [pedAEBfullAborArb]  "pedAEBfullAborArb"
  BrkModStsArb/3(pedAEBfullModStsArb) -> pedAEBfullModStsArb/1, BrkActvArb/3(pedAEBfullModStsArb)
  [cycAEBpartModStsArb] -> cycAEBpartModStsArb/1
  [cycAEBfullModStsArb] -> cycAEBfullModStsArb/1

== system TosAeb/FcwAebJa_Step_sys/ALG_AEBSystem/ALG_AEBController/AEB_StateMachineCalcApp/LatFctStsArb/BrkReadyArb ==
  latAEBModSts : Inport
  brkActvCond : Inport  Port=2
  BrkReadyAborArb : Subsystem
  BrkReadyActvArb : Subsystem
  BrkReadyModStsArb : Subsystem
  BrkReadySuppArb : Subsystem
  Bus Creator : BusCreator  Inputs=6
  Terminator : Terminator
  pedPrefillModStsArb : Outport
  cycPrefillModStsArb : Outport  Port=2
  pedABAModStsArb : Outport  Port=3
  brkReadySuppAborCond : Outport  Port=4
  wires:
  BrkReadySuppArb/1(cycPrefillSuppArb) -> Bus Creator/1  "cycPrefillSuppArb"
  Bus Creator/1 -> brkReadySuppAborCond/1
  BrkReadyAborArb/3(pedABAAborArb) -> Bus Creator/6, [pedABAAborArb]  "pedABAAborArb"
  BrkReadyAborArb/2(cycPrefillAborArb) -> Bus Creator/5, [cycPrefillAborArb]  "cycPrefillAborArb"
  brkActvCond/1 -> BrkReadyAborArb/1(brkActvCond), BrkReadySuppArb/2(brkActvCond)
  latAEBModSts/1 -> BrkReadyModStsArb/1(vehAEBModSts), BrkReadyAborArb/2(latAEBModSts), BrkReadySuppArb/1(latAEBModSts)
  BrkReadySuppArb/2(pedPrefillSuppArb) -> Bus Creator/2  "pedPrefillSuppArb"
  BrkReadySuppArb/3(pedABASuppArb) -> Bus Creator/3  "pedABASuppArb"
  BrkReadyAborArb/1(pedPrefillAborArb) -> [pedPrefillAborArb], Bus Creator/4  "pedPrefillAborArb"
  [cycPrefillAborArb] -> BrkReadyModStsArb/3(cycPrefillAborArb)
  [pedABAAborArb] -> BrkReadyModStsArb/4(pedABAAborArb)
  [pedPrefillAborArb] -> BrkReadyModStsArb/2(pedPrefillAborArb)
  BrkReadyModStsArb/1(pedPrefillModStsArb) -> BrkReadyActvArb/1(prefillModStsArb), [pedPrefillModStsArb]
  BrkReadyModStsArb/2(cycPrefillModStsArb) -> BrkReadyActvArb/2(ABAModStsArb), [cycPrefillModStsArb]
  BrkReadyModStsArb/3(pedABAModStsArb) -> BrkReadyActvArb/3(pedABAModStsArb), pedABAModStsArb/1  "pedABAModStsArb"
  [pedPrefillModStsArb] -> pedPrefillModStsArb/1  "pedPrefillModStsArb"
  [cycPrefillModStsArb] -> cycPrefillModStsArb/1  "cycPrefillModStsArb"
  BrkReadyActvArb/1(brkReadyActv) -> Terminator/1

== system TosAeb/FcwAebJa_Step_sys/ALG_AEBSystem/ALG_AEBController/AEB_StateMachineCalcApp/LatFctStsArb/WarningArb ==
  vehAEBModSts : Inport
  brkActvCond : Inport  Port=2
  Bus Creator : BusCreator  Inputs=2
  Terminator : Terminator
  WarningAborArb : Subsystem
  WarningActvArb : Subsystem
  WarningModStsArb : Subsystem
  WarningSuppArb : Subsystem
  pedPreWarningModStsArb : Outport
  warningSuppAborCond : Outport  Port=2
  wires:
  WarningActvArb/1(pedPreWarningActvCond) -> Terminator/1
  WarningModStsArb/1(pedPreWarningModStsArb) -> pedPreWarningModStsArb/1, WarningActvArb/1(pedPreWarningModStsArb)
  brkActvCond/1 -> WarningAborArb/1(brkActvCond), WarningSuppArb/1(brkActvCond)
  vehAEBModSts/1 -> WarningModStsArb/1(vehAEBModSts)
  Bus Creator/1 -> warningSuppAborCond/1
  WarningSuppArb/1(pedPrewarningSuppArb) -> Bus Creator/2  "pedPrewarningSuppArb"
  WarningAborArb/1(pedPreWarningAborArb) -> WarningModStsArb/2(pedPreWarningAborArb), Bus Creator/1  "pedPreWarningAborArb"

== system TosAeb/FcwAebJa_Step_sys/ALG_AEBSystem/ALG_AEBController/AEB_StateMachineCalcApp/VehAEBStateMachine/ABAStateMachine ==
  inputSignal : Inport
  AEBFuncFaultMap : Subsystem
  AEBStateMachine : AEBSStateMachineLib/AEBStateMachine  SystemSampleTime=-1  VariantActivationTime=update diagram  Latency=0  AutoFrameSizeCalculation=off
  ActivationCondCalc : Subsystem
  ActiveStateAborCondCalc : Subsystem
  Bus Selector : BusSelector  OutputSignals=vehStateCalcInfo.VSC_FCW_AEB_FctSts.FCW_AEB_VehFctSts,vehStateCalcInfo.VSC_AEBFuncSwtSts.AEB_VehResp
  Bus Selector1 : BusSelector  OutputSignals=VehFuncSuppAborCond.vehABASuppCond,vehFctStsArbSuppAborCond.brkReadySuppAborCond.ABABrkReadySuppArb,FuncImpSuppAborCond.brkReadyFuncImpSuppAborCond.brkReadyFuncImpSuppCond.vehABAFuncImpSuppCond
  Bus Selector2 : BusSelector  OutputSignals=VehFuncSuppAborCond.vehABAAborCond,vehFctStsArbSuppAborCond.brkReadySuppAborCond.ABABrkReadyAborArb,FuncImpSuppAborCond.brkReadyFuncImpSuppAborCond.brkReadyFuncImpAborCond.vehABAFuncImpAborCond,FuncManeuAborCond.ABAFuncManeuAborCond
  Constant : Constant  Value=P_AEB_FuncStatus_VehABAWorkTime
  Constant1 : Constant  Value=P_AEB_FuncStatus_VehABAStandstillWorkTime
  Constant2 : Constant  Value=Ts
  Constant3 : Constant  Value=FALSE
  Constant4 : Constant  Value=FALSE
  Constant5 : Constant  Value=FALSE
  Terminator : Terminator
  vehABARawModSts : Outport
  vehABAModSts : Outport  Port=2
  wires:
  AEBStateMachine/2 -> vehABAModSts/1  "vehABAModSts"
  inputSignal/1 -> Bus Selector/1, Bus Selector2/1, Bus Selector1/1
  Bus Selector1/1 -> ActivationCondCalc/1(vehABASuppCond)  "<vehABASuppCond>"
  Bus Selector1/2 -> ActivationCondCalc/2(ABABrkReadySuppArb)  "<ABABrkReadySuppArb>"
  Bus Selector1/3 -> ActivationCondCalc/3(vehABAFuncImpSuppCond)  "<vehABAFuncImpSuppCond>"
  Bus Selector2/1 -> ActiveStateAborCondCalc/1(vehABAAborCond)  "<vehABAAborCond>"
  Bus Selector2/2 -> ActiveStateAborCondCalc/2(ABABrkReadyAborArb)  "<ABABrkReadyAborArb>"
  Bus Selector2/3 -> ActiveStateAborCondCalc/3(vehABAFuncImpAborCond)  "<vehABAFuncImpAborCond>"
  Bus Selector2/4 -> ActiveStateAborCondCalc/4(ABAFuncManeuAborCond)  "<ABAFuncManeuAborCond>"
  AEBStateMachine/3 -> Terminator/1
  AEBStateMachine/1 -> vehABARawModSts/1
  Constant5/1 -> AEBStateMachine/1
  Constant3/1 -> AEBStateMachine/7
  Constant2/1 -> AEBStateMachine/8
  Constant/1 -> AEBStateMachine/9
  Constant1/1 -> AEBStateMachine/10
  Bus Selector/1 -> AEBFuncFaultMap/1(FCW_AEB_VehFctSts)  "<FCW_AEB_VehFctSts>"
  Bus Selector/2 -> AEBStateMachine/3  "<AEB_VehResp>"
  Constant4/1 -> AEBStateMachine/4
  ActivationCondCalc/1(ActivationCondFulfilled) -> AEBStateMachine/5
  ActiveStateAborCondCalc/1(ActiveStateAborCondFulfilled) -> AEBStateMachine/6
  AEBFuncFaultMap/1(Fault) -> AEBStateMachine/2

== system TosAeb/FcwAebJa_Step_sys/ALG_AEBSystem/ALG_AEBController/AEB_StateMachineCalcApp/VehAEBStateMachine/AEBfullStateMachine ==
  inputSignal : Inport
  AEBFuncFaultMap : Subsystem
  AEBStateMachine : AEBSStateMachineLib/AEBStateMachine  SystemSampleTime=-1  VariantActivationTime=update diagram  Latency=0  AutoFrameSizeCalculation=off
  ActivationCondCalc : Subsystem
  ActiveStateAborCondCalc : Subsystem
  Bus Selector : BusSelector  OutputSignals=AVHStsActv,vehStateCalcInfo.VSC_FCW_AEB_FctSts.FCW_AEB_VehFctSts,vehStateCalcInfo.VSC_AEBFuncSwtSts.AEB_VehResp,vehStateCalcInfo.VSCVehStandStill
  Bus Selector1 : BusSelector  OutputSignals=VehFuncSuppAborCond.vehAEBfullSuppCond,vehFctStsArbSuppAborCond.brkSuppAborCondArb.AEBfullSuppArb,FuncImpSuppAborCond.brkFuncImpSuppAborCond.brkFuncImpSuppCond.vehAEBfullFuncImpSuppCond
  Bus Selector2 : BusSelector  OutputSignals=VehFuncSuppAborCond.vehAEBfullAborCondNoStandstill,vehFctStsArbSuppAborCond.brkSuppAborCondArb.AEBfullAborArb,FuncImpSuppAborCond.brkFuncImpSuppAborCond.brkFuncImpAborCond.vehAEBfullFuncImpAborCond,FuncManeuAborCond.vehBrkManeuAborCond
  Bus Selector3 : BusSelector  OutputSignals=VehFuncSuppAborCond.vehAEBpartAborCondStandstill,vehFctStsArbSuppAborCond.brkSuppAborCondArb.AEBfullAborArb,FuncImpSuppAborCond.brkFuncImpSuppAborCond.brkFuncImpAborCond.vehAEBfullFuncImpAborCond,FuncManeuAborCond.vehBrkManeuAborCond
  Constant : Constant  Value=P_AEB_FuncStatus_VehAEBfullWorkTime
  Constant1 : Constant  Value=P_AEB_FuncStatus_VehAEBfullStandstillWorkTime
  Constant2 : Constant  Value=Ts
  StandstillAborCondCalc : Subsystem
  Terminator1 : Terminator
  vehAEBfullRawModSts : Outport
  vehAEBfullModSts : Outport  Port=2
  wires:
  ActivationCondCalc/1(ActivationCondFulfilled) -> AEBStateMachine/5
  StandstillAborCondCalc/1(StandstillAborCondFulfilled) -> AEBStateMachine/7
  ActiveStateAborCondCalc/1(ActiveStateAborCondFulfilled) -> AEBStateMachine/6
  inputSignal/1 -> Bus Selector/1, Bus Selector3/1, Bus Selector2/1, Bus Selector1/1
  Bus Selector1/3 -> ActivationCondCalc/3(vehAEBfullFuncImpSuppCond)  "<vehAEBfullFuncImpSuppCond>"
  Bus Selector1/2 -> ActivationCondCalc/2(AEBfullSuppArb)  "<AEBfullSuppArb>"
  Bus Selector1/1 -> ActivationCondCalc/1(vehAEBfullSuppCond)  "<vehAEBfullSuppCond>"
  Bus Selector2/1 -> ActiveStateAborCondCalc/1(AEBfullAborCondNoStandstill)  "<vehAEBfullAborCondNoStandstill>"
  Bus Selector2/2 -> ActiveStateAborCondCalc/2(AEBfullAborArb)  "<AEBfullAborArb>"
  Bus Selector2/3 -> ActiveStateAborCondCalc/3(vehAEBfullFuncImpAborCond)  "<vehAEBfullFuncImpAborCond>"
  Bus Selector2/4 -> ActiveStateAborCondCalc/4(vehBrkManeuAborCond)  "<vehBrkManeuAborCond>"
  Bus Selector3/1 -> StandstillAborCondCalc/1(AEBfullAborCondStandstill)  "<vehAEBpartAborCondStandstill>"
  Bus Selector3/2 -> StandstillAborCondCalc/2(AEBfullAborArb)  "<AEBfullAborArb>"
  Bus Selector3/3 -> StandstillAborCondCalc/3(vehAEBfullFuncImpAborCond)  "<vehAEBfullFuncImpAborCond>"
  Bus Selector3/4 -> StandstillAborCondCalc/4(vehBrkManeuAborCond)  "<vehBrkManeuAborCond>"
  AEBStateMachine/3 -> Terminator1/1
  AEBStateMachine/2 -> vehAEBfullModSts/1  "vehAEBfullModSts"
  AEBStateMachine/1 -> vehAEBfullRawModSts/1
  Constant2/1 -> AEBStateMachine/8
  Constant/1 -> AEBStateMachine/9
  Constant1/1 -> AEBStateMachine/10
  Bus Selector/2 -> AEBFuncFaultMap/1(FCW_AEB_VehFctSts)  "<FCW_AEB_VehFctSts>"
  Bus Selector/3 -> AEBStateMachine/3  "<AEB_VehResp>"
  Bus Selector/1 -> AEBStateMachine/1  "<AVHStsActv>"
  Bus Selector/4 -> AEBStateMachine/4  "<VSCVehStandStill>"
  AEBFuncFaultMap/1(Fault) -> AEBStateMachine/2

== system TosAeb/FcwAebJa_Step_sys/ALG_AEBSystem/ALG_AEBController/AEB_StateMachineCalcApp/VehAEBStateMachine/AEBpartStateMachine ==
  inputSignal : Inport
  AEBFuncFaultMap : Subsystem
  AEBStateMachine : AEBSStateMachineLib/AEBStateMachine  SystemSampleTime=-1  VariantActivationTime=update diagram  Latency=0  AutoFrameSizeCalculation=off
  ActivationCondCalc : Subsystem
  ActiveStateAborCondCalc : Subsystem
  Bus Selector : BusSelector  OutputSignals=AVHStsActv,vehStateCalcInfo.VSC_FCW_AEB_FctSts.FCW_AEB_VehFctSts,vehStateCalcInfo.VSC_AEBFuncSwtSts.AEB_VehResp,vehStateCalcInfo.VSCVehStandStill
  Bus Selector1 : BusSelector  OutputSignals=VehFuncSuppAborCond.vehAEBpartSuppCond,vehFctStsArbSuppAborCond.brkSuppAborCondArb.AEBpartSuppArb,FuncImpSuppAborCond.brkFuncImpSuppAborCond.brkFuncImpSuppCond.vehAEBpartFuncImpSuppCond
  Bus Selector2 : BusSelector  OutputSignals=VehFuncSuppAborCond.vehAEBpartAborCondNoStandstill,vehFctStsArbSuppAborCond.brkSuppAborCondArb.AEBpartSuppArb,FuncImpSuppAborCond.brkFuncImpSuppAborCond.brkFuncImpAborCond.vehAEBpartFuncImpAborCond,FuncManeuAborCond.vehBrkManeuAborCond
  Bus Selector3 : BusSelector  OutputSignals=VehFuncSuppAborCond.vehAEBpartAborCondStandstill,vehFctStsArbSuppAborCond.brkSuppAborCondArb.AEBpartSuppArb,FuncImpSuppAborCond.brkFuncImpSuppAborCond.brkFuncImpAborCond.vehAEBpartFuncImpAborCond,FuncManeuAborCond.vehBrkManeuAborCond
  Constant : Constant  Value=P_AEB_FuncStatus_VehAEBPartWorkTime
  Constant1 : Constant  Value=P_AEB_FuncStatus_VehAEBPartStandstillWorkTime
  Constant2 : Constant  Value=Ts
  StandstillAborCondCalc : Subsystem
  Terminator : Terminator
  vehAEBpartRawModSts : Outport
  vehAEBpartModSts : Outport  Port=2
  wires:
  ActiveStateAborCondCalc/1(ActiveStateAborCondFulfilled) -> AEBStateMachine/6
  StandstillAborCondCalc/1(StandstillAborCondFulfilled) -> AEBStateMachine/7
  AEBStateMachine/2 -> vehAEBpartModSts/1  "vehAEBpartModSts"
  ActivationCondCalc/1(ActivationCondFulfilled) -> AEBStateMachine/5
  inputSignal/1 -> Bus Selector3/1, Bus Selector2/1, Bus Selector1/1, Bus Selector/1
  Bus Selector1/1 -> ActivationCondCalc/1(vehAEBpartSuppCond)  "<vehAEBpartSuppCond>"
  Bus Selector1/2 -> ActivationCondCalc/2(AEBpartSuppArb)  "<AEBpartSuppArb>"
  Bus Selector1/3 -> ActivationCondCalc/3(vehAEBpartFuncImpSuppCond)  "<vehAEBpartFuncImpSuppCond>"
  Bus Selector2/2 -> ActiveStateAborCondCalc/2(AEBpartSuppArb)  "<AEBpartSuppArb>"
  Bus Selector2/3 -> ActiveStateAborCondCalc/3(vehAEBpartFuncImpAborCond)  "<vehAEBpartFuncImpAborCond>"
  Bus Selector2/1 -> ActiveStateAborCondCalc/1(vehAEBpartAborCondNoStandstill)  "<vehAEBpartAborCondNoStandstill>"
  Bus Selector2/4 -> ActiveStateAborCondCalc/4(vehBrkManeuAborCond)  "<vehBrkManeuAborCond>"
  Bus Selector3/1 -> StandstillAborCondCalc/1(ABEfullAborCondStandstill)  "<vehAEBpartAborCondStandstill>"
  Bus Selector3/2 -> StandstillAborCondCalc/2(BrkFuncAborCond)  "<AEBpartSuppArb>"
  Bus Selector3/3 -> StandstillAborCondCalc/3(brkImpAborCond)  "<vehAEBpartFuncImpAborCond>"
  Bus Selector3/4 -> StandstillAborCondCalc/4(brkImpOpcAborCond)  "<vehBrkManeuAborCond>"
  AEBStateMachine/3 -> Terminator/1
  AEBStateMachine/1 -> vehAEBpartRawModSts/1
  Constant2/1 -> AEBStateMachine/8
  Constant/1 -> AEBStateMachine/9
  Constant1/1 -> AEBStateMachine/10
  Bus Selector/2 -> AEBFuncFaultMap/1(FCW_AEB_VehFctSts)  "<FCW_AEB_VehFctSts>"
  Bus Selector/3 -> AEBStateMachine/3  "<AEB_VehResp>"
  Bus Selector/1 -> AEBStateMachine/1  "<AVHStsActv>"
  Bus Selector/4 -> AEBStateMachine/4  "<VSCVehStandStill>"
  AEBFuncFaultMap/1(Fault) -> AEBStateMachine/2

== system TosAeb/FcwAebJa_Step_sys/ALG_AEBSystem/ALG_AEBController/AEB_StateMachineCalcApp/VehAEBStateMachine/ActuteWarningStateMachine ==
  inputSignal : Inport
  AEBFuncFaultMap : Subsystem
  AEBStateMachine : AEBSStateMachineLib/AEBStateMachine  SystemSampleTime=-1  VariantActivationTime=update diagram  Latency=0  AutoFrameSizeCalculation=off
  ActivationCondCalc : Subsystem
  ActiveStateAborCondCalc : Subsystem
  Bus Selector : BusSelector  OutputSignals=vehStateCalcInfo.VSC_FCW_AEB_FctSts.FCW_AEB_VehFctSts,vehStateCalcInfo.VSC_AEBFuncSwtSts.AEB_VehResp
  Bus Selector1 : BusSelector  OutputSignals=VehFuncSuppAborCond.vehAcuteWarningSuppCond,vehFctStsArbSuppAborCond.warningSuppAborCond.acuteWarningSuppArb,FuncImpSuppAborCond.warningFuncImpSuppAborCond.warningFuncImpSuppCond.vehAcuteWarningFuncimpSuppCond
  Bus Selector2 : BusSelector  OutputSignals=VehFuncSuppAborCond.vehAcuteWarningAborCond,vehFctStsArbSuppAborCond.warningSuppAborCond.acuteWarningSuppArb,FuncImpSuppAborCond.warningFuncImpSuppAborCond.warningFuncImpAborCond.vehAcuteWarningFuncimpAborCond,FuncManeuAborCond.acuteWarningFuncManeuAborCond
  Constant : Constant  Value=P_AEB_FuncStatus_VehAcuteWarningWorkTime
  Constant1 : Constant  Value=P_AEB_FuncStatus_VehAcuteWarningStandstillWorkTime
  Constant2 : Constant  Value=Ts
  Constant3 : Constant  Value=FALSE
  Constant4 : Constant  Value=FALSE
  Constant5 : Constant  Value=FALSE
  Terminator1 : Terminator
  vehAcuteWarningRawModSts : Outport
  vehAcuteWarningModSts : Outport  Port=2
  wires:
  inputSignal/1 -> Bus Selector/1, Bus Selector1/1, Bus Selector2/1
  Bus Selector1/2 -> ActivationCondCalc/2(acuteWarningSuppArb)  "<acuteWarningSuppArb>"
  Bus Selector1/1 -> ActivationCondCalc/1(vehAcuteWarningSuppCond)  "<vehAcuteWarningSuppCond>"
  Bus Selector1/3 -> ActivationCondCalc/3(vehAcuteWarningFuncImpSuppCond)  "<vehAcuteWarningFuncimpSuppCond>"
  Bus Selector2/1 -> ActiveStateAborCondCalc/1(vehAcuteWarningAborCond)  "<vehAcuteWarningAborCond>"
  Bus Selector2/2 -> ActiveStateAborCondCalc/2(acuteWarningAborArb)  "<acuteWarningSuppArb>"
  Bus Selector2/3 -> ActiveStateAborCondCalc/3(vehAcuteWarningFuncImpAborCond)  "<vehAcuteWarningFuncimpAborCond>"
  Bus Selector2/4 -> ActiveStateAborCondCalc/4(acuteWarningFuncManeuAborCond)  "<acuteWarningFuncManeuAborCond>"
  AEBStateMachine/3 -> Terminator1/1
  AEBStateMachine/2 -> vehAcuteWarningModSts/1  "vehAcuteWarningModSts"
  AEBStateMachine/1 -> vehAcuteWarningRawModSts/1
  Constant3/1 -> AEBStateMachine/7
  Constant2/1 -> AEBStateMachine/8
  Constant/1 -> AEBStateMachine/9
  Constant1/1 -> AEBStateMachine/10
  Constant4/1 -> AEBStateMachine/4
  Constant5/1 -> AEBStateMachine/1
  Bus Selector/1 -> AEBFuncFaultMap/1(FCW_AEB_VehFctSts)  "<FCW_AEB_VehFctSts>"
  Bus Selector/2 -> AEBStateMachine/3  "<AEB_VehResp>"
  ActivationCondCalc/1(acuteWarningActivationCondFulfilled) -> AEBStateMachine/5
  ActiveStateAborCondCalc/1(ActiveStateAborCondFulfilled) -> AEBStateMachine/6
  AEBFuncFaultMap/1(Fault) -> AEBStateMachine/2
